simulink model "TosAeb"  (R2021a)
settings: Solver=FixedStepDiscrete  StartTime=0.0  StopTime=inf  FixedStep=0.01  RelTol=1e-3
undefined symbols (not defined in the file or in its folder): ACCModSts, AccSts1, AEB_ObjectMovingOrientation, AEB_ObjectMovingState, CN_BTCActive, CN_CurrentGearR, CN_CurrentGearR_DHT, CN_PTCActive, CN_VDCActive, CN_VSC_EspOff, CN_VSC_FCW_AEB_Error, CN_VSC_FCW_AEB_NoDispay, CN_VSC_FCW_AEB_PerfmDgdtn, CN_VSC_FCW_AEB_TempUnAvlbl, CN_VSC_VehStandstill, CNaccelAvoidMax, CNaccelAvoidMin, CNbrakePedalPress, CNcomfortStrLeftAccelMax, CNcomfortStrLeftAccelMin, CNcomfortStrRightAccelMax, CNcomfortStrRightAccelMin, CNDrvDoorOpen, CNDrvModeEco, CNDrvModeNorm, CNDrvModeOther, CNDrvModeSport, CNDrvSBRreminder, CNNull, CNobjInvalidID, CNPassDoorOpen, CNPassSBRreminder, CNPEBSAccelNegInf, CNPEBSAccelNull, CNPEBSTimeInf, CNPEBSTimeNull, CNSTOPMAX, CNSTOPNULL, CNsubjWidth, CNSwtOFF, CNSwtON, CNTTCMAX, CNTTCNULL, CNVehConfigAEBCycNone, CNVehConfigAEBNone, CNVehConfigAEBPedNone, CNVehConfigFCWNone, CNVehConfigJANone, CollisionSeverity, FALSE, FCWSnvty, JACycObjCalcInfoDefaultParams, JAPedObjCalcInfoDefaultParams, JAVehObjCalcInfoDefaultParams, latCycObjCalcInfoDefaultParams, latPedObjCalcInfoDefaultParams, longObjCalcInfoDefaultParams, ModSts, movingStatus, ObjectType ... (+1112)
libraries: simulink x3031, AEBSFunctionLib x50, Ta_Library x33, AEBSStateMachineLib x23, Mylibrary x12, simulink_extras x7, ObjSelect_lib x3, dspstat3 x2
(part 94 of 102)

== matlab function TosAeb/AEB_Objsel/TA_Processor/AEB_ObjSelect/TargetInfoCheck/Data_precess/TA_EffectCalculate/Obj_FilterFlgCalculate/Long_ObjFilter/OverlapCalculate/BoudingBoxCalculate_Else/PosnatTTI/PredictHostPositionAtTTR/SegmentComponents/SafeDivide/SafeDivide ==
  data: Numerator input; Quotient output; Denominator input; DataTypeMin parameter; DataTypeMax parameter
function Quotient = fcn(Numerator, Denominator, DataTypeMin, DataTypeMax)
    %#codegen
    
    % Test for division by zero and overflow
    if abs(Denominator) > abs(Numerator)*(1/DataTypeMax)
        % Normal divide
        Quotient=Numerator/Denominator;
    else
        % Select output depending on the numerator
        if Numerator>0
            % x/0
            Quotient=DataTypeMax;
        elseif Numerator<0
            % -x/0
            Quotient=DataTypeMin;
        else
            % 0/0
            Quotient=cast(0,class(Numerator));
        end
    end
end

== matlab function TosAeb/AEB_Objsel/TA_Processor/AEB_ObjSelect/TargetInfoCheck/Data_precess/TA_EffectCalculate/Obj_FilterFlgCalculate/Long_ObjFilter/TTR_Calculate/CalculateTTRAndTTM_else/LongitudinalTTRComputer/CalculateTTI/HostAndObjectMoving/CheckIfSolutionExists/LimitedSafeDivide/SafeDivide/SafeDivide ==
  data: Numerator input; Quotient output; Denominator input; DataTypeMin parameter; DataTypeMax parameter
function Quotient = fcn(Numerator, Denominator, DataTypeMin, DataTypeMax)
    %#codegen
    
    % Test for division by zero and overflow
    if abs(Denominator) > abs(Numerator)*(1/DataTypeMax)
        % Normal divide
        Quotient=Numerator/Denominator;
    else
        % Select output depending on the numerator
        if Numerator>0
            % x/0
            Quotient=DataTypeMax;
        elseif Numerator<0
            % -x/0
            Quotient=DataTypeMin;
        else
            % 0/0
            Quotient=cast(0,class(Numerator));
        end
    end
end

== matlab function TosAeb/AEB_Objsel/TA_Processor/AEB_ObjSelect/TargetInfoCheck/Data_precess/TA_EffectCalculate/Obj_FilterFlgCalculate/Long_ObjFilter/TTR_Calculate/CalculateTTRAndTTM_else/LongitudinalTTRComputer/CalculateTTI/HostAndObjectMoving/CheckIfSolutionExists1/LimitedSafeDivide/SafeDivide/SafeDivide ==
  data: Numerator input; Quotient output; Denominator input; DataTypeMin parameter; DataTypeMax parameter
function Quotient = fcn(Numerator, Denominator, DataTypeMin, DataTypeMax)
    %#codegen
    
    % Test for division by zero and overflow
    if abs(Denominator) > abs(Numerator)*(1/DataTypeMax)
        % Normal divide
        Quotient=Numerator/Denominator;
    else
        % Select output depending on the numerator
        if Numerator>0
            % x/0
            Quotient=DataTypeMax;
        elseif Numerator<0
            % -x/0
            Quotient=DataTypeMin;
        else
            % 0/0
            Quotient=cast(0,class(Numerator));
        end
    end
end

== matlab function TosAeb/AEB_Objsel/TA_Processor/AEB_ObjSelect/TargetInfoCheck/Data_precess/TA_EffectCalculate/Obj_FilterFlgCalculate/Long_ObjFilter/TTR_Calculate/CalculateTTRAndTTM_else/LongitudinalTTRComputer/CalculateTTI/HostAndObjectMoving/TimeToIntersectionComputer/QuadraticEquationSolver ==
  data: a input single; x output single; b input single; c input single; SolutionFound output boolean; ZeroThreshold parameter single
function [x, SolutionFound] = fcn(a, b, c, ZeroThreshold)
%#codegen

% Solve ax^2 + bx + c = 0.
% Output x = [x1, x2], where x1 >= x2.

% Case a == 0 and b == 0: Solve c = 0.
aEqualsZero = equalsZero(a, ZeroThreshold);
if aEqualsZero && equalsZero(b, ZeroThreshold)
    
    % Only possible solution exists when also c == 0.
    SolutionFound = equalsZero(c, ZeroThreshold);
    x = single([0; 0]);

% Case a == 0: Solve bx + c = 0.
elseif aEqualsZero
    
    SolutionFound = true;
    x = [-c/b; -c/b];

% Solve the quadratic equation.
% x = (-b ± sqrt(b^2 - 4ac))/(2a).
else
    
    % Complex solutions are not valid.
    bSquared = b*b;
    if 4*a*c > bSquared
        SolutionFound = false;
        x = single([0; 0]);
    else
        SolutionFound = true;
        squareRoot = single(sqrt(bSquared - 4*a*c));
        x1 = (-b + squareRoot)/(2*a); 
        x2 = (-b - squareRoot)/(2*a);
        if x1 > x2
            x = [x1; x2];
        else
            x = [x2; x1];
        end
    end    
end
    
end

% Subfunction equalsZero

function tf = equalsZero(c, ZeroThreshold)

tf = (c < ZeroThreshold && c > -ZeroThreshold);

end

== matlab function TosAeb/AEB_Objsel/TA_Processor/AEB_ObjSelect/TargetInfoCheck/Data_precess/TA_EffectCalculate/Obj_FilterFlgCalculate/Long_ObjFilter/TTR_Calculate/CalculateTTRAndTTM_else/LongitudinalTTRComputer/CalculateTTI/HostAndObjectMoving/TimeToIntersectionComputer1/QuadraticEquationSolver ==
  data: a input single; x output single; b input single; c input single; SolutionFound output boolean; ZeroThreshold parameter single
function [x, SolutionFound] = fcn(a, b, c, ZeroThreshold)
%#codegen

% Solve ax^2 + bx + c = 0.
% Output x = [x1, x2], where x1 >= x2.

% Case a == 0 and b == 0: Solve c = 0.
aEqualsZero = equalsZero(a, ZeroThreshold);
if aEqualsZero && equalsZero(b, ZeroThreshold)
    
    % Only possible solution exists when also c == 0.
    SolutionFound = equalsZero(c, ZeroThreshold);
    x = single([0; 0]);

% Case a == 0: Solve bx + c = 0.
elseif aEqualsZero
    
    SolutionFound = true;
    x = [-c/b; -c/b];

% Solve the quadratic equation.
% x = (-b ± sqrt(b^2 - 4ac))/(2a).
else
    
    % Complex solutions are not valid.
    bSquared = b*b;
    if 4*a*c > bSquared
        SolutionFound = false;
        x = single([0; 0]);
    else
        SolutionFound = true;
        squareRoot = single(sqrt(bSquared - 4*a*c));
        x1 = (-b + squareRoot)/(2*a); 
        x2 = (-b - squareRoot)/(2*a);
        if x1 > x2
            x = [x1; x2];
        else
            x = [x2; x1];
        end
    end    
end
    
end

% Subfunction equalsZero

function tf = equalsZero(c, ZeroThreshold)

tf = (c < ZeroThreshold && c > -ZeroThreshold);

end

== matlab function TosAeb/AEB_Objsel/TA_Processor/AEB_ObjSelect/TargetInfoCheck/Data_precess/TA_EffectCalculate/Obj_FilterFlgCalculate/Long_ObjFilter/TTR_Calculate/CalculateTTRAndTTM_else/LongitudinalTTRComputer/CalculateTTI/StationaryHost/CheckIfSolutionExists/LimitedSafeDivide/SafeDivide/SafeDivide ==
  data: Numerator input; Quotient output; Denominator input; DataTypeMin parameter; DataTypeMax parameter
function Quotient = fcn(Numerator, Denominator, DataTypeMin, DataTypeMax)
    %#codegen
    
    % Test for division by zero and overflow
    if abs(Denominator) > abs(Numerator)*(1/DataTypeMax)
        % Normal divide
        Quotient=Numerator/Denominator;
    else
        % Select output depending on the numerator
        if Numerator>0
            % x/0
            Quotient=DataTypeMax;
        elseif Numerator<0
            % -x/0
            Quotient=DataTypeMin;
        else
            % 0/0
            Quotient=cast(0,class(Numerator));
        end
    end
end

== matlab function TosAeb/AEB_Objsel/TA_Processor/AEB_ObjSelect/TargetInfoCheck/Data_precess/TA_EffectCalculate/Obj_FilterFlgCalculate/Long_ObjFilter/TTR_Calculate/CalculateTTRAndTTM_else/LongitudinalTTRComputer/CalculateTTI/StationaryHost/CheckIfSolutionExists1/LimitedSafeDivide/SafeDivide/SafeDivide ==
  data: Numerator input; Quotient output; Denominator input; DataTypeMin parameter; DataTypeMax parameter
function Quotient = fcn(Numerator, Denominator, DataTypeMin, DataTypeMax)
    %#codegen
    
    % Test for division by zero and overflow
    if abs(Denominator) > abs(Numerator)*(1/DataTypeMax)
        % Normal divide
        Quotient=Numerator/Denominator;
    else
        % Select output depending on the numerator
        if Numerator>0
            % x/0
            Quotient=DataTypeMax;
        elseif Numerator<0
            % -x/0
            Quotient=DataTypeMin;
        else
            % 0/0
            Quotient=cast(0,class(Numerator));
        end
    end
end

== matlab function TosAeb/AEB_Objsel/TA_Processor/AEB_ObjSelect/TargetInfoCheck/Data_precess/TA_EffectCalculate/Obj_FilterFlgCalculate/Long_ObjFilter/TTR_Calculate/CalculateTTRAndTTM_else/LongitudinalTTRComputer/CalculateTTI/StationaryHost/TimeToIntersectionComputer/QuadraticEquationSolver ==
  data: a input single; x output single; b input single; c input single; SolutionFound output boolean; ZeroThreshold parameter single
function [x, SolutionFound] = fcn(a, b, c, ZeroThreshold)
%#codegen

% Solve ax^2 + bx + c = 0.
% Output x = [x1, x2], where x1 >= x2.

% Case a == 0 and b == 0: Solve c = 0.
aEqualsZero = equalsZero(a, ZeroThreshold);
if aEqualsZero && equalsZero(b, ZeroThreshold)
    
    % Only possible solution exists when also c == 0.
    SolutionFound = equalsZero(c, ZeroThreshold);
    x = single([0; 0]);

% Case a == 0: Solve bx + c = 0.
elseif aEqualsZero
    
    SolutionFound = true;
    x = [-c/b; -c/b];

% Solve the quadratic equation.
% x = (-b ± sqrt(b^2 - 4ac))/(2a).
else
    
    % Complex solutions are not valid.
    bSquared = b*b;
    if 4*a*c > bSquared
        SolutionFound = false;
        x = single([0; 0]);
    else
        SolutionFound = true;
        squareRoot = single(sqrt(bSquared - 4*a*c));
        x1 = (-b + squareRoot)/(2*a); 
        x2 = (-b - squareRoot)/(2*a);
        if x1 > x2
            x = [x1; x2];
        else
            x = [x2; x1];
        end
    end    
end
    
end

% Subfunction equalsZero

function tf = equalsZero(c, ZeroThreshold)

tf = (c < ZeroThreshold && c > -ZeroThreshold);

end

== matlab function TosAeb/AEB_Objsel/TA_Processor/AEB_ObjSelect/TargetInfoCheck/Data_precess/TA_EffectCalculate/Obj_FilterFlgCalculate/Long_ObjFilter/TTR_Calculate/CalculateTTRAndTTM_else/LongitudinalTTRComputer/CalculateTTI/StationaryHost/TimeToIntersectionComputer1/QuadraticEquationSolver ==
  data: a input single; x output single; b input single; c input single; SolutionFound output boolean; ZeroThreshold parameter single
function [x, SolutionFound] = fcn(a, b, c, ZeroThreshold)
%#codegen

% Solve ax^2 + bx + c = 0.
% Output x = [x1, x2], where x1 >= x2.

% Case a == 0 and b == 0: Solve c = 0.
aEqualsZero = equalsZero(a, ZeroThreshold);
if aEqualsZero && equalsZero(b, ZeroThreshold)
    
    % Only possible solution exists when also c == 0.
    SolutionFound = equalsZero(c, ZeroThreshold);
    x = single([0; 0]);

% Case a == 0: Solve bx + c = 0.
elseif aEqualsZero
    
    SolutionFound = true;
    x = [-c/b; -c/b];

% Solve the quadratic equation.
% x = (-b ± sqrt(b^2 - 4ac))/(2a).
else
    
    % Complex solutions are not valid.
    bSquared = b*b;
    if 4*a*c > bSquared
        SolutionFound = false;
        x = single([0; 0]);
    else
        SolutionFound = true;
        squareRoot = single(sqrt(bSquared - 4*a*c));
        x1 = (-b + squareRoot)/(2*a); 
        x2 = (-b - squareRoot)/(2*a);
        if x1 > x2
            x = [x1; x2];
        else
            x = [x2; x1];
        end
    end    
end
    
end

% Subfunction equalsZero

function tf = equalsZero(c, ZeroThreshold)

tf = (c < ZeroThreshold && c > -ZeroThreshold);

end

== matlab function TosAeb/AEB_Objsel/TA_Processor/AEB_ObjSelect/TargetInfoCheck/Data_precess/TA_EffectCalculate/Obj_FilterFlgCalculate/Long_ObjFilter/TTR_Calculate/CalculateTTRAndTTM_else/LongitudinalTTRComputer/CalculateTTI/StationaryObject/CheckIfSolutionExists/LimitedSafeDivide/SafeDivide/SafeDivide ==
  data: Numerator input; Quotient output; Denominator input; DataTypeMin parameter; DataTypeMax parameter
function Quotient = fcn(Numerator, Denominator, DataTypeMin, DataTypeMax)
    %#codegen
    
    % Test for division by zero and overflow
    if abs(Denominator) > abs(Numerator)*(1/DataTypeMax)
        % Normal divide
        Quotient=Numerator/Denominator;
    else
        % Select output depending on the numerator
        if Numerator>0
            % x/0
            Quotient=DataTypeMax;
        elseif Numerator<0
            % -x/0
            Quotient=DataTypeMin;
        else
            % 0/0
            Quotient=cast(0,class(Numerator));
        end
    end
end

== matlab function TosAeb/AEB_Objsel/TA_Processor/AEB_ObjSelect/TargetInfoCheck/Data_precess/TA_EffectCalculate/Obj_FilterFlgCalculate/Long_ObjFilter/TTR_Calculate/CalculateTTRAndTTM_else/LongitudinalTTRComputer/CalculateTTI/StationaryObject/CheckIfSolutionExists1/LimitedSafeDivide/SafeDivide/SafeDivide ==
  data: Numerator input; Quotient output; Denominator input; DataTypeMin parameter; DataTypeMax parameter
function Quotient = fcn(Numerator, Denominator, DataTypeMin, DataTypeMax)
    %#codegen
    
    % Test for division by zero and overflow
    if abs(Denominator) > abs(Numerator)*(1/DataTypeMax)
        % Normal divide
        Quotient=Numerator/Denominator;
    else
        % Select output depending on the numerator
        if Numerator>0
            % x/0
            Quotient=DataTypeMax;
        elseif Numerator<0
            % -x/0
            Quotient=DataTypeMin;
        else
            % 0/0
            Quotient=cast(0,class(Numerator));
        end
    end
end

== matlab function TosAeb/AEB_Objsel/TA_Processor/AEB_ObjSelect/TargetInfoCheck/Data_precess/TA_EffectCalculate/Obj_FilterFlgCalculate/Long_ObjFilter/TTR_Calculate/CalculateTTRAndTTM_else/LongitudinalTTRComputer/CalculateTTI/StationaryObject/TimeToIntersectionComputer/QuadraticEquationSolver ==
  data: a input single; x output single; b input single; c input single; SolutionFound output boolean; ZeroThreshold parameter single
function [x, SolutionFound] = fcn(a, b, c, ZeroThreshold)
%#codegen

% Solve ax^2 + bx + c = 0.
% Output x = [x1, x2], where x1 >= x2.

% Case a == 0 and b == 0: Solve c = 0.
aEqualsZero = equalsZero(a, ZeroThreshold);
if aEqualsZero && equalsZero(b, ZeroThreshold)
    
    % Only possible solution exists when also c == 0.
    SolutionFound = equalsZero(c, ZeroThreshold);
    x = single([0; 0]);

% Case a == 0: Solve bx + c = 0.
elseif aEqualsZero
    
    SolutionFound = true;
    x = [-c/b; -c/b];

% Solve the quadratic equation.
% x = (-b ± sqrt(b^2 - 4ac))/(2a).
else
    
    % Complex solutions are not valid.
    bSquared = b*b;
    if 4*a*c > bSquared
        SolutionFound = false;
        x = single([0; 0]);
    else
        SolutionFound = true;
        squareRoot = single(sqrt(bSquared - 4*a*c));
        x1 = (-b + squareRoot)/(2*a); 
        x2 = (-b - squareRoot)/(2*a);
        if x1 > x2
            x = [x1; x2];
        else
            x = [x2; x1];
        end
    end    
end
    
end

% Subfunction equalsZero

function tf = equalsZero(c, ZeroThreshold)

tf = (c < ZeroThreshold && c > -ZeroThreshold);

end

== matlab function TosAeb/AEB_Objsel/TA_Processor/AEB_ObjSelect/TargetInfoCheck/Data_precess/TA_EffectCalculate/Obj_FilterFlgCalculate/Long_ObjFilter/TTR_Calculate/CalculateTTRAndTTM_else/LongitudinalTTRComputer/CalculateTTI/StationaryObject/TimeToIntersectionComputer1/QuadraticEquationSolver ==
  data: a input single; x output single; b input single; c input single; SolutionFound output boolean; ZeroThreshold parameter single
function [x, SolutionFound] = fcn(a, b, c, ZeroThreshold)
%#codegen

% Solve ax^2 + bx + c = 0.
% Output x = [x1, x2], where x1 >= x2.

% Case a == 0 and b == 0: Solve c = 0.
aEqualsZero = equalsZero(a, ZeroThreshold);
if aEqualsZero && equalsZero(b, ZeroThreshold)
    
    % Only possible solution exists when also c == 0.
    SolutionFound = equalsZero(c, ZeroThreshold);
    x = single([0; 0]);

% Case a == 0: Solve bx + c = 0.
elseif aEqualsZero
    
    SolutionFound = true;
    x = [-c/b; -c/b];

% Solve the quadratic equation.
% x = (-b ± sqrt(b^2 - 4ac))/(2a).
else
    
    % Complex solutions are not valid.
    bSquared = b*b;
    if 4*a*c > bSquared
        SolutionFound = false;
        x = single([0; 0]);
    else
        SolutionFound = true;
        squareRoot = single(sqrt(bSquared - 4*a*c));
        x1 = (-b + squareRoot)/(2*a); 
        x2 = (-b - squareRoot)/(2*a);
        if x1 > x2
            x = [x1; x2];
        else
            x = [x2; x1];
        end
    end    
end
    
end

% Subfunction equalsZero

function tf = equalsZero(c, ZeroThreshold)

tf = (c < ZeroThreshold && c > -ZeroThreshold);

end

== matlab function TosAeb/AEB_Objsel/TA_Processor/AEB_ObjSelect/TargetInfoCheck/Data_precess/TA_EffectCalculate/Obj_FilterFlgCalculate/Long_ObjFilter/TTR_Calculate/CalculateTTRAndTTM_else/Obj_AbsInfo/StoppingDataForTTICalculation/LimitedSafeDivide/SafeDivide/SafeDivide ==
  data: Numerator input; Quotient output; Denominator input; DataTypeMin parameter; DataTypeMax parameter
function Quotient = fcn(Numerator, Denominator, DataTypeMin, DataTypeMax)
    %#codegen
    
    % Test for division by zero and overflow
    if abs(Denominator) > abs(Numerator)*(1/DataTypeMax)
        % Normal divide
        Quotient=Numerator/Denominator;
    else
        % Select output depending on the numerator
        if Numerator>0
            % x/0
            Quotient=DataTypeMax;
        elseif Numerator<0
            % -x/0
            Quotient=DataTypeMin;
        else
            % 0/0
            Quotient=cast(0,class(Numerator));
        end
    end
end

== matlab function TosAeb/AEB_Objsel/TA_Processor/AEB_ObjSelect/TargetInfoCheck/Data_precess/TA_EffectCalculate/Obj_FilterFlgCalculate/Long_ObjFilter/TTR_Calculate/CalculateTTRAndTTM_else/Obj_AbsInfo/StoppingDataForTTICalculation/LimitedSafeDivide1/SafeDivide/SafeDivide ==
  data: Numerator input; Quotient output; Denominator input; DataTypeMin parameter; DataTypeMax parameter
function Quotient = fcn(Numerator, Denominator, DataTypeMin, DataTypeMax)
    %#codegen
    
    % Test for division by zero and overflow
    if abs(Denominator) > abs(Numerator)*(1/DataTypeMax)
        % Normal divide
        Quotient=Numerator/Denominator;
    else
        % Select output depending on the numerator
        if Numerator>0
            % x/0
            Quotient=DataTypeMax;
        elseif Numerator<0
            % -x/0
            Quotient=DataTypeMin;
        else
            % 0/0
            Quotient=cast(0,class(Numerator));
        end
    end
end

== stateflow chart TosAeb/AEB_Objsel/TA_Processor/AEB_ObjSelect/TargetInfoCheck/Data_precess/TA_EffectCalculate/Obj_FilterFlgCalculate/Long_ObjFilter/TTR_Calculate/CalculateTTRAndTTM_else/Subsystem/Chart1 ==
  state Deactive
    en:
    TTI_Flg = true;
    du:
    TTI_Flg = true;
  state Active
    en:
    TTI_Flg = false;
    du:
    TTI_Flg = false;
  (default) -> Deactive
  Deactive -> Active : [
      | Flg == false &&...
      |  TTI >= P_TA_TTIValidNumber + single(0.5)
      | ]
  Active -> Deactive : [
      | Flg == true &&...
      |  TTI <= P_TA_TTIValidNumber
      | ]
  data: Flg input; TTI input; P_TA_TTIValidNumber input; TTI_Flg output boolean

== matlab function TosAeb/AEB_Objsel/TA_Processor/AEB_ObjSelect/TargetInfoCheck/Data_precess/TA_EffectCalculate/Obj_FilterFlgCalculate/WeightCoef_Cal/WeightCoef_Cal1 ==
  data: Utility input; Obj_WeightCoef output; Overlap_Current input; Overlap_Prediction input
function Obj_WeightCoef = fcn(Utility,Overlap_Current,Overlap_Prediction)
%设置有效目标对应数值为"0"，无效目标的为255，本车道目标为"1",预测本车道目标为"2"
%当目标为本车道目标，同时也是预测本车道目标时，设置数值为"3"
weightCoef = uint8(zeros(1,32));
for i = 1:32
%对有效目标对应数值进行处理，避免混淆
    if Utility(i) == false
        Utility(i) = uint8(255);
    else
       Utility(i) = uint8(0);
    end
%对同一目标权重进行重新赋值
    if Utility(i) == uint8(0) 
        if Overlap_Current == true && Overlap_Prediction == false
            weightCoef(i) = uint8(1);
        elseif Overlap_Current == false && Overlap_Prediction == true
             weightCoef(i) = uint8(2);
        elseif Overlap_Current == true && Overlap_Prediction == true
            weightCoef(i) = uint8(3);
        else
            weightCoef(i) = Utility(i);
        end
    else
         weightCoef(i) = Utility(i);
    end
end
Obj_WeightCoef = weightCoef;
end

== matlab function TosAeb/AEB_Objsel/TA_Processor/AEB_ObjSelect/TargetInfoCheck/Data_precess/TA_EffectCalculate/ValideateObj/ObjPos_Calculate/ObjPos_Calculateelse/InPath_Cal/DetermineInPath/LimitedSafeDivide/SafeDivide/SafeDivide ==
  data: Numerator input; Quotient output; Denominator input; DataTypeMin parameter; DataTypeMax parameter
function Quotient = fcn(Numerator, Denominator, DataTypeMin, DataTypeMax)
    %#codegen
    
    % Test for division by zero and overflow
    if abs(Denominator) > abs(Numerator)*(1/DataTypeMax)
        % Normal divide
        Quotient=Numerator/Denominator;
    else
        % Select output depending on the numerator
        if Numerator>0
            % x/0
            Quotient=DataTypeMax;
        elseif Numerator<0
            % -x/0
            Quotient=DataTypeMin;
        else
            % 0/0
            Quotient=cast(0,class(Numerator));
        end
    end
end

== matlab function TosAeb/AEB_Objsel/TA_Processor/AEB_ObjSelect/TargetInfoCheck/Data_precess/TA_EffectCalculate/ValideateObj/ObjPos_Calculate/ObjPos_Calculateelse/InPath_Cal/DetermineInPath/PredictHostLateralPositionAtTTR/CalculateHeading/ExtractVelocitiesAtStartOfSegments/ExtractVelocityVectorFirstPoint/PositionAndDerivative ==
  data: P0 input; x output single; P1 input; P2 input; P3 input; tau input; dx output single
function [x,dx] = fcn(P0,P1,P2,P3,tau)
%#codegen

%TODO Description is missing 

%For performance reasons the original expression, below, has been rewritten  

%Original expressions 
% dx为x的对自变量tau的导数
% 式中x为距离，dx为速度
% 式中tau为x取值对应的概率值，p0，p1,p2,p3前系数值的和为"1"
%x=(1-tau)^3*P0 + 3*(1-tau)^2*tau*P1 + 3*(1-tau)*tau.^2*P2 + tau^3*P3;
%dx = -3*(1-tau)^2*P0 + 3*(3*tau^2 - 4*tau + 1)*P1 + 3*(2*tau - 3*tau^2)*P2 + 3*tau^2*P3; 

tauPov2 = tau*tau; 
tauPov3 = tau*tauPov2; 
x = (3*(P1 - P2) - P0 + P3)*tauPov3 + 3*(P0 - 2*P1 + P2)*tauPov2 + 3*(P1 - P0)*tau + P0; 
dx = (9*P1 - 3*P0 - 9*P2 + 3*P3)*tauPov2 + (6*P0 - 12*P1 + 6*P2)*tau - 3*P0 + 3*P1;

== matlab function TosAeb/AEB_Objsel/TA_Processor/AEB_ObjSelect/TargetInfoCheck/Data_precess/TA_EffectCalculate/ValideateObj/ObjPos_Calculate/ObjPos_Calculateelse/InPath_Cal/DetermineInPath/PredictHostLateralPositionAtTTR/CalculateHeading/ExtractVelocitiesAtStartOfSegments/ExtractVelocityVectorFirstPoint/PositionAndDerivative1 ==
  data: P0 input; y output single; P1 input; P2 input; P3 input; tau input; dy output single
function [y, dy] = fun(P0,P1,P2,P3,tau)
%#codegen

%TODO Description is missing 

%For performance reasons the original expression, below, has been rewritten  

%Original expressions 
% dx为x的对自变量tau的导数
% 式中x为距离，dx为速度
% 式中tau为x取值对应的概率值，p0，p1,p2,p3前系数值的和为"1"
%x=(1-tau)^3*P0 + 3*(1-tau)^2*tau*P1 + 3*(1-tau)*tau.^2*P2 + tau^3*P3;
%dx = -3*(1-tau)^2*P0 + 3*(3*tau^2 - 4*tau + 1)*P1 + 3*(2*tau - 3*tau^2)*P2 + 3*tau^2*P3; 

tauPov2 = tau*tau; 
tauPov3 = tau*tauPov2; 
y = (3*(P1 - P2) - P0 + P3)*tauPov3 + 3*(P0 - 2*P1 + P2)*tauPov2 + 3*(P1 - P0)*tau + P0; 
dy = (9*P1 - 3*P0 - 9*P2 + 3*P3)*tauPov2 + (6*P0 - 12*P1 + 6*P2)*tau - 3*P0 + 3*P1;

== matlab function TosAeb/AEB_Objsel/TA_Processor/AEB_ObjSelect/TargetInfoCheck/Data_precess/TA_EffectCalculate/ValideateObj/ObjPos_Calculate/ObjPos_Calculateelse/InPath_Cal/DetermineInPath/PredictHostLateralPositionAtTTR/CalculateHeading/FindValidIndex ==
  data: SegmentIndex input; SpeedValid input; ValidSegmentIndex output
function ValidSegmentIndex = fcn(SegmentIndex, SpeedValid)
%#codegen

% Searches for segment start points with detectable speed.
% The highest such index that is not further than the current segment is
% returned. Zero means that no such index were found.

% Default value of the found index.
ValidSegmentIndex = uint8(0);

for i = uint8(1:size(SpeedValid,1))
    % Loop through all indices and search for detectable speed.
    if SpeedValid(i) && i<=SegmentIndex
        % Speed is detectable and we have not searched further than the
        % current segment.
        ValidSegmentIndex = uint8(i);
    end
end
end

== matlab function TosAeb/AEB_Objsel/TA_Processor/AEB_ObjSelect/TargetInfoCheck/Data_precess/TA_EffectCalculate/ValideateObj/ObjPos_Calculate/ObjPos_Calculateelse/InPath_Cal/DetermineInPath/PredictHostLateralPositionAtTTR/CalculateHeading/FindValidVelocityVector ==
  data: ValidVelocityY output; CurrentVelocityValid input; ValidVelocityX output; ValidSegmentIndex input; CurrentVelocityX input; CurrentVelocityY input; SegmentVelocitiesX input; SegmentVelocitiesY input
function [ValidVelocityY, ValidVelocityX] = fcn(CurrentVelocityValid, ValidSegmentIndex, CurrentVelocityX, CurrentVelocityY, SegmentVelocitiesX, SegmentVelocitiesY)
%#codegen

if CurrentVelocityValid
    % The current position in the segments has detectable speed.
    % Use that speed vector to estimate heading angle.
    ValidVelocityX = CurrentVelocityX;
    ValidVelocityY = CurrentVelocityY;
elseif ValidSegmentIndex > 0
    % An earlier start point of a segment has detectable speed.
    % Use the speed vector of the most recent start point with detectable
    % speed.
    ValidVelocityX = SegmentVelocitiesX(ValidSegmentIndex);
    ValidVelocityY = SegmentVelocitiesY(ValidSegmentIndex);
else
    % No valid speed has been detected.
    % Use speed vector that creates zero heading angle.
    % If we don't move, no heading change is needed.
    ValidVelocityX = single(0);
    ValidVelocityY = single(0);
end

end

== matlab function TosAeb/AEB_Objsel/TA_Processor/AEB_ObjSelect/TargetInfoCheck/Data_precess/TA_EffectCalculate/ValideateObj/ObjPos_Calculate/ObjPos_Calculateelse/InPath_Cal/DetermineInPath/PredictHostLateralPositionAtTTR/PositionAndDerivative ==
  data: P0 input; x output single; P1 input; P2 input; P3 input; tau input; dx output single
function [x,dx] = fcn(P0,P1,P2,P3,tau)
%#codegen

%TODO Description is missing 

%For performance reasons the original expression, below, has been rewritten  

%Original expressions 
% dx为x的对自变量tau的导数
% 式中x为距离，dx为速度
% 式中tau为x取值对应的概率值，p0，p1,p2,p3前系数值的和为"1"
%x=(1-tau)^3*P0 + 3*(1-tau)^2*tau*P1 + 3*(1-tau)*tau.^2*P2 + tau^3*P3;
%dx = -3*(1-tau)^2*P0 + 3*(3*tau^2 - 4*tau + 1)*P1 + 3*(2*tau - 3*tau^2)*P2 + 3*tau^2*P3; 

tauPov2 = tau*tau; 
tauPov3 = tau*tauPov2; 
x = (3*(P1 - P2) - P0 + P3)*tauPov3 + 3*(P0 - 2*P1 + P2)*tauPov2 + 3*(P1 - P0)*tau + P0; 
dx = (9*P1 - 3*P0 - 9*P2 + 3*P3)*tauPov2 + (6*P0 - 12*P1 + 6*P2)*tau - 3*P0 + 3*P1;

== matlab function TosAeb/AEB_Objsel/TA_Processor/AEB_ObjSelect/TargetInfoCheck/Data_precess/TA_EffectCalculate/ValideateObj/ObjPos_Calculate/ObjPos_Calculateelse/InPath_Cal/DetermineInPath/PredictHostLateralPositionAtTTR/PositionAndDerivative1 ==
  data: P0 input; y output single; P1 input; P2 input; P3 input; tau input; dy output single
function [y, dy] = fun(P0,P1,P2,P3,tau)
%#codegen

%TODO Description is missing 

%For performance reasons the original expression, below, has been rewritten  

%Original expressions 
% dx为x的对自变量tau的导数
% 式中x为距离，dx为速度
% 式中tau为x取值对应的概率值，p0，p1,p2,p3前系数值的和为"1"
%x=(1-tau)^3*P0 + 3*(1-tau)^2*tau*P1 + 3*(1-tau)*tau.^2*P2 + tau^3*P3;
%dx = -3*(1-tau)^2*P0 + 3*(3*tau^2 - 4*tau + 1)*P1 + 3*(2*tau - 3*tau^2)*P2 + 3*tau^2*P3; 

tauPov2 = tau*tau; 
tauPov3 = tau*tauPov2; 
y = (3*(P1 - P2) - P0 + P3)*tauPov3 + 3*(P0 - 2*P1 + P2)*tauPov2 + 3*(P1 - P0)*tau + P0; 
dy = (9*P1 - 3*P0 - 9*P2 + 3*P3)*tauPov2 + (6*P0 - 12*P1 + 6*P2)*tau - 3*P0 + 3*P1;

== matlab function TosAeb/AEB_Objsel/TA_Processor/AEB_ObjSelect/TargetInfoCheck/Data_precess/TA_EffectCalculate/ValideateObj/ObjPos_Calculate/ObjPos_Calculateelse/InPath_Cal/DetermineInPath/PredictHostLateralPositionAtTTR/SegmentComponents/SafeDivide/SafeDivide ==
  data: Numerator input; Quotient output; Denominator input; DataTypeMin parameter; DataTypeMax parameter
function Quotient = fcn(Numerator, Denominator, DataTypeMin, DataTypeMax)
    %#codegen
    
    % Test for division by zero and overflow
    if abs(Denominator) > abs(Numerator)*(1/DataTypeMax)
        % Normal divide
        Quotient=Numerator/Denominator;
    else
        % Select output depending on the numerator
        if Numerator>0
            % x/0
            Quotient=DataTypeMax;
        elseif Numerator<0
            % -x/0
            Quotient=DataTypeMin;
        else
            % 0/0
            Quotient=cast(0,class(Numerator));
        end
    end
end

== matlab function TosAeb/AEB_Objsel/TA_Processor/AEB_ObjSelect/TargetInfoCheck/Data_precess/TA_EffectCalculate/ValideateObj/ObjPos_Calculate/ObjPos_Calculateelse/InPath_Cal/Position_InPath ==
  data: Overlap_Flg output; Obj_Lenth input; Obj_Width input; Obj_Dx input; Overlap_Rate output; Obj_Dy input; Obj_vy input; Obj_Heading input; Subj_Width input; P_TA_PrmForObjDisThrd input; Overlap_delay input
 function [Overlap_Flg,Overlap_Rate]  = fcn(Obj_Lenth,Obj_Width,Obj_Dx,Obj_Dy,Obj_vy,Obj_Heading,Subj_Width,P_TA_PrmForObjDisThrd,Overlap_delay)
%自车左右侧横坐标及目标车左右横坐标，不考虑Heanding角
Subj_R_Width = Subj_Width/single(2);
Subj_L_Width = -Subj_Width/single(2);
Obj_R_Dy = Obj_Dy + Obj_Width/single(2);
Obj_L_Dy = Obj_Dy - Obj_Width/single(2);

    %目标车左右侧横坐标,考虑Heanding角,计算目标与自车dy为两中心位置
    if Obj_Heading >= single(0) && Obj_Heading < single(0.75) && abs(Obj_vy) > single(0)
        Obj_R_Dy = Obj_Dy + Obj_Width/single(2) + Obj_Lenth * sin(Obj_Heading);
        Obj_L_Dy = Obj_Dy - Obj_Width/single(2) - Obj_Lenth * sin(Obj_Heading);
    elseif Obj_Heading < single(0) && Obj_Heading > single(-0.75) && abs(Obj_vy) > single(0)
        Obj_R_Dy = Obj_Dy + Obj_Width/single(2) - Obj_Lenth * sin(Obj_Heading);
        Obj_L_Dy = Obj_Dy - Obj_Width/single(2) + Obj_Lenth * sin(Obj_Heading);
    else
    end

    %目标车左右侧横坐标,考虑Heanding角,计算目标与自车dy为最近点位置
%     if Obj_Heading >= single(0) && Obj_Heading < single(0.75) && abs(Obj_vy) > single(0)
%         Obj_R_Dy = Obj_Dy + Obj_Width* cos(Obj_Heading);
%         Obj_L_Dy = Obj_Dy;
%     elseif Obj_Heading < single(0) && Obj_Heading > single(-0.75) && abs(Obj_vy) > single(0)
%         Obj_R_Dy = Obj_Dy;
%         Obj_L_Dy = Obj_Dy - Obj_Width* cos(Obj_Heading);
%     else
%     end

if Obj_Dx >= single(P_TA_PrmForObjDisThrd)
    if Obj_R_Dy <= Subj_L_Width || Obj_L_Dy >= Subj_R_Width
        Overlap_Rate = single(0);
    else
        if Obj_R_Dy >= Subj_R_Width
            Overlap_Rate = (Subj_R_Width - Obj_L_Dy)/Subj_Width;
        else
            Overlap_Rate = (Obj_R_Dy - Subj_L_Width)/Subj_Width;
        end
    end
    if Subj_R_Width >= Obj_R_Dy && Subj_L_Width <= Obj_L_Dy
        Overlap_Rate = single(1);
    else
    end
else
    Overlap_Rate = single(0);
end
if Overlap_Rate < single(0)
    Overlap_Rate = single(0);
end
Overlap_Rate = min(Overlap_Rate,single(1));
if Overlap_Rate > single(0.15) %&& Overlap_delay > single(0.12)
    Overlap_Flg = true;
else
    Overlap_Flg = false;
end
end

== matlab function TosAeb/AEB_Objsel/TA_Processor/AEB_ObjSelect/TargetSelect/JA_ObjSelect/MATLAB Function ==
  data: ID input; TTC input; SortNum output; CllsnFlg input; VaildID output
function [SortNum,VaildID] = fcn(ID,TTC,CllsnFlg)

temp_id = uint8(0);
temp_num = uint8(16);
for i=1:16
    if TTC(i)<=single(2)&&CllsnFlg(i)==true
       temp_id=ID(i);
       temp_num=uint8(i);
       break
    else
       temp_id=uint8(0);
       temp_num=uint8(16);
    end
end

SortNum = temp_num;
VaildID = temp_id;

== stateflow chart TosAeb/AEB_Objsel/TA_Processor/AEB_ObjSelect/TargetSelect/Lat_ObjSelect/AEB_LatObj/AEB_LatTAelse/Lat_TASelect ==
  (default) -> (junction 25) : {
      | num_Vecter = Init_Num;
      | ID_Vecter = Init_ID;
      | Length = uint8(0);
      | i = uint8(0);
      | }
  (junction 25) -> (junction 26) [order 1] : [sumLat_ObjFlg>= uint8(10)]
  (junction 25) -> (junction 41) [order 2]
  (junction 26) -> (junction 36) : {
      | k = uint8(0);
      | }
  (junction 41) -> (junction 39) [order 1] : [i < uint8(32)]
  (junction 39) -> (junction 40) [order 1] : [
      | Obj_Valid[i] == true &&...
      | Lat_ObjIntersect[i] == true &&...
      |  Obj_ID[i] ~= uint8(0)
      | ]
  (junction 42) -> (junction 41) : {
      | i++;
      | }
  (junction 41) -> (junction 60) [order 2] : {
      | j = uint8(0);
      | }
  (junction 39) -> (junction 42) [order 2] : {
      | num_Vecter;
      | ID_Vecter;
      | Length;
      | }
  (junction 40) -> (junction 51) : {
      | num_Vecter[Length] = i + uint8(1);
      | ID_Vecter[Length] = Obj_ID[i];
      | Length = Length + uint8(1);
      | }
  (junction 51) -> (junction 42)
  (junction 36) -> (junction 52) [order 1] : [k < uint8(10)]
  (junction 54) -> (junction 36) : {
      | k++;
      | i++;
      | }
  (junction 36) -> (junction 57) [order 2]
  (junction 52) -> (junction 54) : {
      | num_Vecter[Length] = Sort[i];
      | ID_Vecter[Length] = Obj_ID[Sort[i]];
      | Length = Length + uint8(1);
      | }
  (junction 60) -> (junction 62) [order 1] : [j < uint8(10)]
  (junction 62) -> (junction 70) [order 1] : [Obj_Valid[j] == true &&...
      | Lat_ObjIntersect[j] == false &&...
      |  Obj_ID[j] ~= uint8(0)]
  (junction 70) -> (junction 75) [order 1] : [Length > uint8(9)]
  (junction 64) -> (junction 60) : {
      | j++;
      | }
  (junction 60) -> (junction 67) [order 2]
  (junction 70) -> (junction 71) [order 2] : {
      | num_Vecter[Length] = j + uint8(1);
      | ID_Vecter[Length] = Obj_ID[j];
      | Length = Length + uint8(1);
      | }
  (junction 75) -> (junction 77) : {
      | num_Vecter;
      | ID_Vecter;
      | Length;
      | }
  (junction 62) -> (junction 64) [order 2] : {
      | num_Vecter;
      | ID_Vecter;
      | Length;
      | }
  (junction 71) -> (junction 64)
  (junction 77) -> (junction 71)
  (junction 57) -> (junction 67)
  (junction 67) -> (junction 46) : {
      | num = num_Vecter;
      | ID = ID_Vecter;
      | }
  data: Obj_Valid input; Obj_ID input; sumLat_ObjFlg input; Lat_ObjIntersect input; Length local uint8; i local uint8; num output uint8 size=10; ID output uint8 size=10; ID_Vecter local single size=10; num_Vecter local single size=10; Sort input; Init_ID input uint8 size=10; Init_Num input uint8 size=10; j local double; k local double

== stateflow chart TosAeb/AEB_Objsel/TA_Processor/AEB_ObjSelect/TargetSelect/Lat_ObjSelect/AEB_LatObj/AEB_LatTAif/Lat_TASelect ==
  (default) -> (junction 1) : {
      | num_Vecter = Init_Num;
      | ID_Vecter = Init_ID;
      | Length = uint8(0);
      | i = uint8(0);
      | }
  (junction 1) -> (junction 4) [order 1] : [i < uint8(32)]
  (junction 4) -> (junction 10) [order 1] : [
      | Obj_Valid[i] == true &&...
      |  Obj_ID[i] ~= uint8(0)
      | ]
  (junction 3) -> (junction 1) : {
      | i++;
      | }
  (junction 1) -> (junction 6) [order 2] : {
      | num = num_Vecter;
      | ID = ID_Vecter;
      | }
  (junction 4) -> (junction 3) [order 2]
  (junction 10) -> (junction 12) : {
      | num_Vecter[Length] = i + uint8(1);
      | ID_Vecter[Length] = Obj_ID[i];
      | Length = Length + uint8(1);
      | }
  (junction 12) -> (junction 3)
  data: Obj_Valid input; Obj_ID input; Length local uint8; i local uint8; num output uint8 size=10; ID output uint8 size=10; ID_Vecter local single size=10; num_Vecter local single size=10; Init_Num input uint8 size=10; Init_ID input uint8 size=10

== matlab function TosAeb/AEB_Objsel/TA_Processor/AEB_ObjSelect/TargetSelect/Long_ObjSelect/Obj_IDConfirm/Target_Select_decle/Obj_select ==
  data: re_Valid input; Obj_ID input; sort_dx input; dy input; Obj_vx input; ValidTarget_ID output; Obj_state input; Obj_Orentation input; Subj_vx input; Veh_AEBFunState input; Boudingbox_Calculate input; Obj_Coef input; TTI input; AccTgtIdn input; Overlap_Rate input; P_TA_PrmForObjSpdStationary input; ID_num output; long_TTC output
function [ValidTarget_ID,ID_num,long_TTC] = fcn(re_Valid,Obj_ID,sort_dx,dy,...
    Obj_vx,Obj_state,Obj_Orentation,Subj_vx,Veh_AEBFunState,Boudingbox_Calculate,Obj_Coef,TTI,AccTgtIdn,Overlap_Rate,P_TA_PrmForObjSpdStationary)
num = uint8(255);
temp_ID = uint8(0);
% 判断是否存在有效目标
if sum(re_Valid) == uint8(0)
    ValidTarget_ID1 = uint8(255);
    ID_num1 = num;
else
    for i = 1:32
        ii = sort_dx(i);
        %判断目标的有效性
      
        if (Obj_Coef(ii) == uint8(3) ||Obj_Coef(ii) == uint8(1))&& abs(dy(ii)) < single(3)
            num = uint8(ii);
            temp_ID = Obj_ID(ii);  
            break;
        elseif Obj_Coef(ii) == uint8(255) ||Obj_Coef(ii) == uint8(0)|| (TTI(ii) >= single(90)&&Veh_AEBFunState==0) || TTI(ii) <= single(-6) || (Overlap_Rate(ii) ==false && (Obj_state(ii) ~= uint8(2) && Obj_state(ii) ~= uint8(3)))
            continue;
        else
            %判断目标运动状态，是否满足ACC目标选择条件
            if Obj_vx(ii) + Subj_vx >= P_TA_PrmForObjSpdStationary && Obj_state(ii) ~= uint8(2) && Obj_Orentation(ii) ~= uint8(2) && Obj_Orentation(ii) ~= uint8(3) && Obj_state(ii) ~= uint8(0) && AccTgtIdn == Obj_ID(ii)
                num = uint8(ii);
                temp_ID = Obj_ID(ii);
                break;
            elseif Boudingbox_Calculate(ii) == true&&Subj_vx<=single(2.77)&&Obj_ID(ii) ~= uint8(0) && Obj_state(ii) ~= uint8(0) && TTI(ii) ~= single(100) 
                    num = uint8(ii);
                    temp_ID = Obj_ID(ii);
            elseif  Subj_vx>single(2.77)&&Obj_ID(ii) ~= uint8(0) && Obj_state(ii) ~= uint8(0) && TTI(ii) ~= single(100)   
                    num = uint8(ii);
                    temp_ID = Obj_ID(ii);
                    break;
            else
                    num = uint8(255);
                    temp_ID = uint8(0);
            end
        end
    end 
   ValidTarget_ID1 = temp_ID;
   ID_num1 = num;
end
 ValidTarget_ID = ValidTarget_ID1;
    ID_num = ID_num1;
    long_TTC = TTI;

== stateflow chart TosAeb/AEB_Objsel/TA_Processor/PreProcessor/ObjectPrecessor/TAObjInfoCalc/For Each Subsystem/ForEachInput/PosErrorCalc/FakeObjJudge ==
  state ErrorObj
    en:
    CurentID = ID;
    du:
    ConfFlg=false;
  state Initialization
    ConfFlg=true;
  (default) -> Initialization
  ErrorObj -> Initialization : [FakeFlg==false&&ID~=CurentID...
      | &&ID~=LastID]
  Initialization -> ErrorObj : [FakeFlg==true]
  data: ID input; LastID input; ConfFlg output; FakeFlg input; CurentID local

== matlab function TosAeb/AEB_Objsel/TA_Processor/PreProcessor/ObjectPrecessor/TAObjInfoCalc/For Each Subsystem/Info_Check1/Basic_Info_check/DxCorrect1/MATLAB Function1 ==
  data: range input; R_filtered output; rangeRate input; rangeAccel input; targetChanged input; targetPresent input; RR_filtered output
function [R_filtered,RR_filtered]  = rangerangeRateKalmanFilter(range, rangeRate, rangeAccel, targetChanged, targetPresent)


persistent x P;
% Initialize state variables 
if isempty(x) 
   x = cast([range; rangeRate], class(range));
   P =  cast([1 0; 0 1], class(range));
end

% Reset filter conditions
if x(1) - range > 1 || targetChanged || targetPresent == 0
   x = cast([range; rangeRate], class(range));
   P =  cast([1 0; 0 1], class(range));
end

if targetPresent ~=0

T = cast(0.02, class(range));

A = cast([1, T; 0, 1], class(range));  % State transition matrix 输入矩阵
B = cast([0.5 * T^2; T], class(range));    % Input matrix 输入矩阵
 
% Measurement matrix not included in the equations for now since it is unitary
% If this changes it needs to be included in the update step
% C = [1 0;
%      0 1];  % Measurement matrix  测量矩阵% 
 
R = cast([2^2,    0;
          0,      0.3^2], class(range)); % measurment covariance matrix 测量噪声 传感器特性

Q = cast([0.1^2,  0;
          0,      0.1^2], class(range)); % process covariance matrix 过程噪声 车辆颠簸造成的误差
 
y = [range; rangeRate]; % measurement vector 测量数据
 
% Prediction update  预测更新
x = A*x + B * rangeAccel;    
P = A*P*A' + Q;  %先验状态估计误差协方差状态矩阵            

% Measurement update
S=(P+R);

% Calculate determinant 计算矩阵的值
detS = (S(1,1) * S(2,2) - S(1,2) * S(2,1));

% Safe divider
if detS < 0.001 && detS > -0.001
    if detS > 0
        detS = cast(0.001, class(range));
    else
        detS = cast(-0.001, class(range));
    end
end

% Invert matrix
Sinv = [S(2,2) -S(1,2); -S(2,1) S(1,1)]./detS;%二阶矩阵的求法口诀：主对角线元素互换，副对角线元素变号 [3]。

% Update
Mn = P * Sinv;
x = x + Mn * (y - x);  %MN为卡尔曼增益     
P = (eye(2) - Mn) * P;  
end
R_filtered = x(1);
RR_filtered = x(2);

== matlab function TosAeb/AEB_Objsel/TA_Processor/PreProcessor/ObjectPrecessor/TAObjInfoCalc/For Each Subsystem/Info_Check1/LaneAssign_check/LaneAssign_Cal ==
  data: ID_Change input; Fus_ObjLaneAssign output; ObsID_u8 input; ObsLaneAssign_u8 input; ObsPosX_s input; ObsPosY_s input; P_TA_PrmForLaneWidthThred input
function Fus_ObjLaneAssign = fcn(ID_Change,ObsID_u8,ObsLaneAssign_u8,ObsPosX_s,ObsPosY_s,P_TA_PrmForLaneWidthThred)
%目标所在车道
%0x0: UNKNOWN 0x1: LEFT_LEFT 0x2: LEFT 0x3: HOST 0x4: RIGHT  0x5: RIGHT_RIGHT
ObsLaneAssign = uint8(0);
%检查目标是否有效
if (ID_Change == true || ObsID_u8 == uint8(0)) && ObsPosX_s > single(0)
    ObsLaneAssign = uint8(0);
else
    if ObsLaneAssign_u8 == uint8(0)
        if abs(ObsPosY_s) <= single(0.5*P_TA_PrmForLaneWidthThred)
            ObsLaneAssign = uint8(3);
        elseif ObsPosY_s <= single(1.5*P_TA_PrmForLaneWidthThred) && ObsPosY_s>= single(0.5*P_TA_PrmForLaneWidthThred)
            ObsLaneAssign = uint8(2);
        elseif ObsPosY_s >= single(1.5*P_TA_PrmForLaneWidthThred) && ObsPosY_s<= single(2.5*P_TA_PrmForLaneWidthThred)
            ObsLaneAssign = uint8(1);
        elseif ObsPosY_s >= single(-1.5*P_TA_PrmForLaneWidthThred) && ObsPosY_s<= single(-0.5*P_TA_PrmForLaneWidthThred)
            ObsLaneAssign = uint8(4);
        elseif ObsPosY_s <= single(-1.5*P_TA_PrmForLaneWidthThred) && ObsPosY_s>= single(-2.5*P_TA_PrmForLaneWidthThred)
            ObsLaneAssign = uint8(5);
        else
            ObsLaneAssign = uint8(0);
        end
    else
        ObsLaneAssign = ObsLaneAssign_u8;
    end
end
Fus_ObjLaneAssign = ObsLaneAssign;
end
